simulink model "TosAeb"  (R2021a)
settings: Solver=FixedStepDiscrete  StartTime=0.0  StopTime=inf  FixedStep=0.01  RelTol=1e-3
undefined symbols (not defined in the file or in its folder): ACCModSts, AccSts1, AEB_ObjectMovingOrientation, AEB_ObjectMovingState, CN_BTCActive, CN_CurrentGearR, CN_CurrentGearR_DHT, CN_PTCActive, CN_VDCActive, CN_VSC_EspOff, CN_VSC_FCW_AEB_Error, CN_VSC_FCW_AEB_NoDispay, CN_VSC_FCW_AEB_PerfmDgdtn, CN_VSC_FCW_AEB_TempUnAvlbl, CN_VSC_VehStandstill, CNaccelAvoidMax, CNaccelAvoidMin, CNbrakePedalPress, CNcomfortStrLeftAccelMax, CNcomfortStrLeftAccelMin, CNcomfortStrRightAccelMax, CNcomfortStrRightAccelMin, CNDrvDoorOpen, CNDrvModeEco, CNDrvModeNorm, CNDrvModeOther, CNDrvModeSport, CNDrvSBRreminder, CNNull, CNobjInvalidID, CNPassDoorOpen, CNPassSBRreminder, CNPEBSAccelNegInf, CNPEBSAccelNull, CNPEBSTimeInf, CNPEBSTimeNull, CNSTOPMAX, CNSTOPNULL, CNsubjWidth, CNSwtOFF, CNSwtON, CNTTCMAX, CNTTCNULL, CNVehConfigAEBCycNone, CNVehConfigAEBNone, CNVehConfigAEBPedNone, CNVehConfigFCWNone, CNVehConfigJANone, CollisionSeverity, FALSE, FCWSnvty, JACycObjCalcInfoDefaultParams, JAPedObjCalcInfoDefaultParams, JAVehObjCalcInfoDefaultParams, latCycObjCalcInfoDefaultParams, latPedObjCalcInfoDefaultParams, longObjCalcInfoDefaultParams, ModSts, movingStatus, ObjectType ... (+1112)
libraries: simulink x3031, AEBSFunctionLib x50, Ta_Library x33, AEBSStateMachineLib x23, Mylibrary x12, simulink_extras x7, ObjSelect_lib x3, dspstat3 x2
(part 32 of 102)

== system TosAeb/FcwAebJa_Step_sys/ALG_AEBSystem/ALG_AEBController/AEB_StateMachineCalcApp/LatAEBStateMachine/pedPrefillStateMachine/ActivationCondCalc ==
  pedPrefillSuppCond : Inport
  pedPrefillSuppArb : Inport  Port=2
  pedPrefillFuncImpSuppCond : Inport  Port=3
  Compare To Constant : simulink/Logic and Bit Operations/Compare To Constant  relop===  const=FALSE  OutDataTypeStr=boolean
  Compare To Constant1 : simulink/Logic and Bit Operations/Compare To Constant  relop===  const=FALSE  OutDataTypeStr=boolean
  Compare To Constant2 : simulink/Logic and Bit Operations/Compare To Constant  relop===  const=FALSE  OutDataTypeStr=boolean
  Logical Operator : Logic  Inputs=3  AllPortsSameDT=off  OutDataTypeStr=boolean
  ActivationCondFulfilled : Outport
  wires:
  pedPrefillSuppCond/1 -> Compare To Constant/1
  pedPrefillSuppArb/1 -> Compare To Constant1/1
  pedPrefillFuncImpSuppCond/1 -> Compare To Constant2/1
  Compare To Constant1/1 -> Logical Operator/2
  Compare To Constant/1 -> Logical Operator/1
  Compare To Constant2/1 -> Logical Operator/3
  Logical Operator/1 -> ActivationCondFulfilled/1

== system TosAeb/FcwAebJa_Step_sys/ALG_AEBSystem/ALG_AEBController/AEB_StateMachineCalcApp/LatAEBStateMachine/pedPrefillStateMachine/ActiveStateAborCondCalc ==
  pedPrefillAborCond : Inport
  pedPrefillAborArb : Inport  Port=2
  pedPrefillFuncImpAborCond : Inport  Port=3
  vehBrkManeuAborCond : Inport  Port=4
  Compare To Constant{,1..3} (4 alike) : simulink/Logic and Bit Operations/Compare To Constant  relop===  const=TRUE  OutDataTypeStr=boolean
  Logical Operator : Logic  Operator=OR  Inputs=4  AllPortsSameDT=off  OutDataTypeStr=boolean
  ActiveStateAborCondFulfilled : Outport
  wires:
  Logical Operator/1 -> ActiveStateAborCondFulfilled/1
  Compare To Constant2/1 -> Logical Operator/3
  Compare To Constant/1 -> Logical Operator/1
  Compare To Constant1/1 -> Logical Operator/2
  pedPrefillFuncImpAborCond/1 -> Compare To Constant2/1
  pedPrefillAborArb/1 -> Compare To Constant1/1
  pedPrefillAborCond/1 -> Compare To Constant/1
  vehBrkManeuAborCond/1 -> Compare To Constant3/1
  Compare To Constant3/1 -> Logical Operator/4

== system TosAeb/FcwAebJa_Step_sys/ALG_AEBSystem/ALG_AEBController/AEB_StateMachineCalcApp/LatFctStsArb/BrkArb/BrkAborArb ==
  vehAEBModSts : Inport
  Bus Selector : BusSelector  OutputSignals=cycAEBfullModSts,pedAEBfullModSts
  Compare To Constant2 : simulink/Logic and Bit Operations/Compare To Constant  relop===  const=ModSts.ActiveState  OutDataTypeStr=boolean
  Compare To Constant3 : simulink/Logic and Bit Operations/Compare To Constant  relop===  const=ModSts.ActiveState  OutDataTypeStr=boolean
  Constant : Constant  Value=FALSE  OutDataTypeStr=boolean
  Logical Operator : Logic  Operator=OR  AllPortsSameDT=off  OutDataTypeStr=boolean
  cycAEBpartAborArb : Outport
  cycAEBfullAborArb : Outport  Port=2
  pedAEBfullAborArb : Outport  Port=3
  wires:
  Constant/1 -> pedAEBfullAborArb/1
  vehAEBModSts/1 -> Bus Selector/1
  Compare To Constant3/1 -> Logical Operator/2, cycAEBfullAborArb/1
  Compare To Constant2/1 -> Logical Operator/1
  Logical Operator/1 -> cycAEBpartAborArb/1
  Bus Selector/1 -> Compare To Constant2/1  "<cycAEBfullModSts>"
  Bus Selector/2 -> Compare To Constant3/1  "<pedAEBfullModSts>"

== system TosAeb/FcwAebJa_Step_sys/ALG_AEBSystem/ALG_AEBController/AEB_StateMachineCalcApp/LatFctStsArb/BrkArb/BrkActvArb ==
  cycAEBpartModStsArb : Inport
  cycAEBfullModStsArb : Inport  Port=2
  pedAEBfullModStsArb : Inport  Port=3
  Compare To Constant : simulink/Logic and Bit Operations/Compare To Constant  relop===  const=ModSts.ActiveState  OutDataTypeStr=boolean
  Compare To Constant1 : simulink/Logic and Bit Operations/Compare To Constant  relop===  const=ModSts.ActiveState  OutDataTypeStr=boolean
  Compare To Constant2 : simulink/Logic and Bit Operations/Compare To Constant  relop===  const=ModSts.ActiveState  OutDataTypeStr=boolean
  Logical Operator : Logic  Operator=OR  Inputs=3  AllPortsSameDT=off  OutDataTypeStr=boolean
  brkActvCond : Outport
  wires:
  pedAEBfullModStsArb/1 -> Compare To Constant2/1
  cycAEBfullModStsArb/1 -> Compare To Constant1/1
  cycAEBpartModStsArb/1 -> Compare To Constant/1
  Logical Operator/1 -> brkActvCond/1
  Compare To Constant/1 -> Logical Operator/1
  Compare To Constant1/1 -> Logical Operator/2
  Compare To Constant2/1 -> Logical Operator/3

== system TosAeb/FcwAebJa_Step_sys/ALG_AEBSystem/ALG_AEBController/AEB_StateMachineCalcApp/LatFctStsArb/BrkArb/BrkModStsArb ==
  vehAEBModSts : Inport
  cycAEBpartAborArb : Inport  Port=2
  cycAEBfullAborArb : Inport  Port=3
  pedAEBfullAborArb : Inport  Port=4
  Bus Selector : BusSelector  OutputSignals=cycAEBpartModSts
  Bus Selector1 : BusSelector  OutputSignals=cycAEBfullModSts
  Bus Selector2 : BusSelector  OutputSignals=pedAEBfullModSts
  Compare To Constant : simulink/Logic and Bit Operations/Compare To Constant  relop=~=  const=ModSts.ActiveState  OutDataTypeStr=boolean
  Compare To Constant1 : simulink/Logic and Bit Operations/Compare To Constant  relop===  const=FALSE  OutDataTypeStr=boolean
  Compare To Constant2 : simulink/Logic and Bit Operations/Compare To Constant  relop=~=  const=ModSts.ActiveState  OutDataTypeStr=boolean
  Compare To Constant3 : simulink/Logic and Bit Operations/Compare To Constant  relop===  const=FALSE  OutDataTypeStr=boolean
  Compare To Constant4 : simulink/Logic and Bit Operations/Compare To Constant  relop=~=  const=ModSts.ActiveState  OutDataTypeStr=boolean
  Compare To Constant5 : simulink/Logic and Bit Operations/Compare To Constant  relop===  const=FALSE  OutDataTypeStr=boolean
  Constant : Constant  Value=ModSts.StandbyState
  Constant1 : Constant  Value=ModSts.StandbyState
  Constant2 : Constant  Value=ModSts.StandbyState
  Switch{,1..5} (6 alike) : Switch  Criteria=u2 ~= 0
  cycAEBpartModStsArb : Outport
  cycAEBfullModStsArb : Outport  Port=2
  pedAEBfullModStsArb : Outport  Port=3
  wires:
  vehAEBModSts/1 -> Bus Selector/1, Bus Selector2/1, Bus Selector1/1
  Switch2/1 -> cycAEBfullModStsArb/1
  Switch3/1 -> Switch2/3
  Constant1/1 -> Switch3/3
  Compare To Constant3/1 -> Switch3/2
  cycAEBfullAborArb/1 -> Compare To Constant3/1
  Compare To Constant2/1 -> Switch2/2
  Bus Selector1/1 -> Compare To Constant2/1, Switch3/1, Switch2/1  "<cycAEBfullModSts>"
  Switch/1 -> cycAEBpartModStsArb/1
  Constant/1 -> Switch1/3
  cycAEBpartAborArb/1 -> Compare To Constant1/1
  Compare To Constant1/1 -> Switch1/2
  Switch1/1 -> Switch/3
  Bus Selector/1 -> Compare To Constant/1, Switch1/1, Switch/1  "<cycAEBpartModSts>"
  Compare To Constant/1 -> Switch/2
  Switch4/1 -> pedAEBfullModStsArb/1
  pedAEBfullAborArb/1 -> Compare To Constant5/1
  Bus Selector2/1 -> Compare To Constant4/1, Switch5/1, Switch4/1  "<pedAEBfullModSts>"
  Compare To Constant4/1 -> Switch4/2
  Compare To Constant5/1 -> Switch5/2
  Constant2/1 -> Switch5/3
  Switch5/1 -> Switch4/3

== system TosAeb/FcwAebJa_Step_sys/ALG_AEBSystem/ALG_AEBController/AEB_StateMachineCalcApp/LatFctStsArb/BrkArb/BrkSuppArb ==
  vehAEBModSts : Inport
  Bus Selector : BusSelector  OutputSignals=cycAEBfullModSts,pedAEBfullModSts
  Compare To Constant2 : simulink/Logic and Bit Operations/Compare To Constant  relop===  const=ModSts.ActiveState  OutDataTypeStr=boolean
  Compare To Constant6 : simulink/Logic and Bit Operations/Compare To Constant  relop===  const=ModSts.ActiveState  OutDataTypeStr=boolean
  Constant : Constant  Value=FALSE
  Logical Operator : Logic  Operator=OR  AllPortsSameDT=off  OutDataTypeStr=boolean
  cycAEBpartSuppArb : Outport
  cycAEBfullSuppArb : Outport  Port=2
  pedAEBfullSuppArb : Outport  Port=3
  wires:
  Constant/1 -> pedAEBfullSuppArb/1
  Compare To Constant2/1 -> Logical Operator/2, cycAEBfullSuppArb/1
  Compare To Constant6/1 -> Logical Operator/1
  vehAEBModSts/1 -> Bus Selector/1
  Logical Operator/1 -> cycAEBpartSuppArb/1
  Bus Selector/1 -> Compare To Constant6/1  "<cycAEBfullModSts>"
  Bus Selector/2 -> Compare To Constant2/1  "<pedAEBfullModSts>"

== system TosAeb/FcwAebJa_Step_sys/ALG_AEBSystem/ALG_AEBController/AEB_StateMachineCalcApp/LatFctStsArb/BrkReadyArb/BrkReadyAborArb ==
  brkActvCond : Inport
  latAEBModSts : Inport  Port=2
  Bus Selector : BusSelector  OutputSignals=pedPrefillModSts
  Compare To Constant : simulink/Logic and Bit Operations/Compare To Constant  relop===  const=TRUE  OutDataTypeStr=boolean
  Compare To Constant2 : simulink/Logic and Bit Operations/Compare To Constant  relop===  const=ModSts.ActiveState  OutDataTypeStr=boolean
  Constant : Constant  Value=FALSE  OutDataTypeStr=boolean
  Logical Operator : Logic  Operator=OR  AllPortsSameDT=off  OutDataTypeStr=boolean
  pedPrefillAborArb : Outport
  cycPrefillAborArb : Outport  Port=2
  pedABAAborArb : Outport  Port=3
  wires:
  brkActvCond/1 -> Compare To Constant/1
  Constant/1 -> pedABAAborArb/1
  latAEBModSts/1 -> Bus Selector/1
  Bus Selector/1 -> Compare To Constant2/1  "<pedPrefillModSts>"
  Compare To Constant/1 -> Logical Operator/1, pedPrefillAborArb/1
  Compare To Constant2/1 -> Logical Operator/2
  Logical Operator/1 -> cycPrefillAborArb/1

== system TosAeb/FcwAebJa_Step_sys/ALG_AEBSystem/ALG_AEBController/AEB_StateMachineCalcApp/LatFctStsArb/BrkReadyArb/BrkReadyActvArb ==
  prefillModStsArb : Inport
  ABAModStsArb : Inport  Port=2
  pedABAModStsArb : Inport  Port=3
  Compare To Constant : simulink/Logic and Bit Operations/Compare To Constant  relop===  const=ModSts.ActiveState  OutDataTypeStr=boolean
  Compare To Constant1 : simulink/Logic and Bit Operations/Compare To Constant  relop===  const=ModSts.ActiveState  OutDataTypeStr=boolean
  Compare To Constant2 : simulink/Logic and Bit Operations/Compare To Constant  relop===  const=ModSts.ActiveState  OutDataTypeStr=boolean
  Logical Operator : Logic  Operator=OR  Inputs=3  AllPortsSameDT=off  OutDataTypeStr=boolean
  brkReadyActv : Outport
  wires:
  ABAModStsArb/1 -> Compare To Constant1/1
  Logical Operator/1 -> brkReadyActv/1
  prefillModStsArb/1 -> Compare To Constant/1
  pedABAModStsArb/1 -> Compare To Constant2/1
  Compare To Constant/1 -> Logical Operator/1
  Compare To Constant1/1 -> Logical Operator/2
  Compare To Constant2/1 -> Logical Operator/3

== system TosAeb/FcwAebJa_Step_sys/ALG_AEBSystem/ALG_AEBController/AEB_StateMachineCalcApp/LatFctStsArb/BrkReadyArb/BrkReadyModStsArb ==
  vehAEBModSts : Inport
  pedPrefillAborArb : Inport  Port=2
  cycPrefillAborArb : Inport  Port=3
  pedABAAborArb : Inport  Port=4
  Bus Selector : BusSelector  OutputSignals=pedPrefillModSts
  Bus Selector1 : BusSelector  OutputSignals=cycPrefillModSts
  Bus Selector2 : BusSelector  OutputSignals=pedABAModSts
  Compare To Constant1 : simulink/Logic and Bit Operations/Compare To Constant  relop=~=  const=ModSts.ActiveState  OutDataTypeStr=boolean
  Compare To Constant2 : simulink/Logic and Bit Operations/Compare To Constant  relop=~=  const=ModSts.ActiveState  OutDataTypeStr=boolean
  Compare To Constant3 : simulink/Logic and Bit Operations/Compare To Constant  relop===  const=FALSE  OutDataTypeStr=boolean
  Compare To Constant4 : simulink/Logic and Bit Operations/Compare To Constant  relop=~=  const=ModSts.ActiveState  OutDataTypeStr=boolean
  Compare To Constant5 : simulink/Logic and Bit Operations/Compare To Constant  relop===  const=FALSE  OutDataTypeStr=boolean
  Compare To Constant6 : simulink/Logic and Bit Operations/Compare To Constant  relop===  const=FALSE  OutDataTypeStr=boolean
  Constant1 : Constant  Value=ModSts.StandbyState
  Constant2 : Constant  Value=ModSts.StandbyState
  Constant3 : Constant  Value=ModSts.StandbyState
  Switch{1..6} (6 alike) : Switch  Criteria=u2 ~= 0
  pedPrefillModStsArb : Outport
  cycPrefillModStsArb : Outport  Port=2
  pedABAModStsArb : Outport  Port=3
  wires:
  Switch2/1 -> cycPrefillModStsArb/1  "cycPrefillModStsArb"
  Switch3/1 -> Switch2/3
  Constant1/1 -> Switch3/3
  Compare To Constant3/1 -> Switch3/2
  cycPrefillAborArb/1 -> Compare To Constant3/1
  Compare To Constant2/1 -> Switch2/2
  Bus Selector1/1 -> Compare To Constant2/1, Switch2/1, Switch3/1  "<cycPrefillModSts>"
  Switch1/1 -> pedPrefillModStsArb/1  "pedPrefillModStsArb"
  vehAEBModSts/1 -> Bus Selector/1, Bus Selector2/1, Bus Selector1/1
  Bus Selector2/1 -> Switch5/1, Switch4/1, Compare To Constant4/1  "<pedABAModSts>"
  Compare To Constant4/1 -> Switch4/2
  pedABAAborArb/1 -> Compare To Constant5/1
  Compare To Constant5/1 -> Switch5/2
  Constant2/1 -> Switch5/3
  Switch5/1 -> Switch4/3
  Switch4/1 -> pedABAModStsArb/1
  pedPrefillAborArb/1 -> Compare To Constant6/1
  Compare To Constant1/1 -> Switch1/2
  Constant3/1 -> Switch6/3
  Switch6/1 -> Switch1/3
  Bus Selector/1 -> Switch1/1, Switch6/1, Compare To Constant1/1  "<pedPrefillModSts>"
  Compare To Constant6/1 -> Switch6/2

== system TosAeb/FcwAebJa_Step_sys/ALG_AEBSystem/ALG_AEBController/AEB_StateMachineCalcApp/LatFctStsArb/BrkReadyArb/BrkReadySuppArb ==
  latAEBModSts : Inport
  brkActvCond : Inport  Port=2
  Bus Selector : BusSelector  OutputSignals=pedPrefillModSts
  Compare To Constant : simulink/Logic and Bit Operations/Compare To Constant  relop===  const=TRUE  OutDataTypeStr=boolean
  Compare To Constant1 : simulink/Logic and Bit Operations/Compare To Constant  relop===  const=ModSts.ActiveState  OutDataTypeStr=boolean
  Constant : Constant  Value=FALSE  OutDataTypeStr=boolean
  Logical Operator : Logic  Operator=OR  AllPortsSameDT=off  OutDataTypeStr=boolean
  cycPrefillSuppArb : Outport
  pedPrefillSuppArb : Outport  Port=2
  pedABASuppArb : Outport  Port=3
  wires:
  Constant/1 -> pedABASuppArb/1
  brkActvCond/1 -> Compare To Constant/1
  latAEBModSts/1 -> Bus Selector/1
  Bus Selector/1 -> Compare To Constant1/1  "<pedPrefillModSts>"
  Compare To Constant1/1 -> Logical Operator/1
  Compare To Constant/1 -> Logical Operator/2, pedPrefillSuppArb/1
  Logical Operator/1 -> cycPrefillSuppArb/1

== system TosAeb/FcwAebJa_Step_sys/ALG_AEBSystem/ALG_AEBController/AEB_StateMachineCalcApp/LatFctStsArb/WarningArb/WarningAborArb ==
  brkActvCond : Inport
  Compare To Constant5 : simulink/Logic and Bit Operations/Compare To Constant  relop===  const=TRUE  OutDataTypeStr=boolean
  pedPreWarningAborArb : Outport
  wires:
  Compare To Constant5/1 -> pedPreWarningAborArb/1
  brkActvCond/1 -> Compare To Constant5/1

== system TosAeb/FcwAebJa_Step_sys/ALG_AEBSystem/ALG_AEBController/AEB_StateMachineCalcApp/LatFctStsArb/WarningArb/WarningActvArb ==
  pedPreWarningModStsArb : Inport
  Compare To Constant : simulink/Logic and Bit Operations/Compare To Constant  relop===  const=ModSts.ActiveState  OutDataTypeStr=boolean
  pedPreWarningActvCond : Outport
  wires:
  pedPreWarningModStsArb/1 -> Compare To Constant/1
  Compare To Constant/1 -> pedPreWarningActvCond/1

== system TosAeb/FcwAebJa_Step_sys/ALG_AEBSystem/ALG_AEBController/AEB_StateMachineCalcApp/LatFctStsArb/WarningArb/WarningModStsArb ==
  vehAEBModSts : Inport
  pedPreWarningAborArb : Inport  Port=2
  Bus Selector : BusSelector  OutputSignals=pedPreWarningModSts
  Compare To Constant : simulink/Logic and Bit Operations/Compare To Constant  relop=~=  const=ModSts.ActiveState  OutDataTypeStr=boolean
  Compare To Constant1 : simulink/Logic and Bit Operations/Compare To Constant  relop===  const=FALSE  OutDataTypeStr=boolean
  Constant : Constant  Value=ModSts.StandbyState
  Switch : Switch  Criteria=u2 ~= 0
  Switch1 : Switch  Criteria=u2 ~= 0
  pedPreWarningModStsArb : Outport
  wires:
  vehAEBModSts/1 -> Bus Selector/1
  Bus Selector/1 -> Switch/1, Switch1/1, Compare To Constant/1  "<pedPreWarningModSts>"
  Compare To Constant/1 -> Switch/2
  Switch1/1 -> Switch/3
  Compare To Constant1/1 -> Switch1/2
  pedPreWarningAborArb/1 -> Compare To Constant1/1
  Constant/1 -> Switch1/3
  Switch/1 -> pedPreWarningModStsArb/1

== system TosAeb/FcwAebJa_Step_sys/ALG_AEBSystem/ALG_AEBController/AEB_StateMachineCalcApp/LatFctStsArb/WarningArb/WarningSuppArb ==
  brkActvCond : Inport
  Compare To Constant4 : simulink/Logic and Bit Operations/Compare To Constant  relop===  const=TRUE  OutDataTypeStr=boolean
  pedPrewarningSuppArb : Outport
  wires:
  Compare To Constant4/1 -> pedPrewarningSuppArb/1
  brkActvCond/1 -> Compare To Constant4/1

== system TosAeb/FcwAebJa_Step_sys/ALG_AEBSystem/ALG_AEBController/AEB_StateMachineCalcApp/VehAEBStateMachine/ABAStateMachine/AEBFuncFaultMap ==
  FCW_AEB_VehFctSts : Inport
  Compare To Constant : simulink/Logic and Bit Operations/Compare To Constant  relop===  const=CN_VSC_FCW_AEB_Error  OutDataTypeStr=boolean
  Fault : Outport
  wires:
  FCW_AEB_VehFctSts/1 -> Compare To Constant/1
  Compare To Constant/1 -> Fault/1

== system TosAeb/FcwAebJa_Step_sys/ALG_AEBSystem/ALG_AEBController/AEB_StateMachineCalcApp/VehAEBStateMachine/ABAStateMachine/ActivationCondCalc ==
  vehABASuppCond : Inport
  ABABrkReadySuppArb : Inport  Port=2
  vehABAFuncImpSuppCond : Inport  Port=3
  Compare To Constant : simulink/Logic and Bit Operations/Compare To Constant  relop===  const=FALSE  OutDataTypeStr=boolean
  Compare To Constant1 : simulink/Logic and Bit Operations/Compare To Constant  relop===  const=FALSE  OutDataTypeStr=boolean
  Compare To Constant2 : simulink/Logic and Bit Operations/Compare To Constant  relop===  const=FALSE  OutDataTypeStr=boolean
  Logical Operator : Logic  Inputs=3  AllPortsSameDT=off  OutDataTypeStr=boolean
  ActivationCondFulfilled : Outport
  wires:
  vehABASuppCond/1 -> Compare To Constant/1
  ABABrkReadySuppArb/1 -> Compare To Constant1/1
  vehABAFuncImpSuppCond/1 -> Compare To Constant2/1
  Compare To Constant1/1 -> Logical Operator/2
  Compare To Constant/1 -> Logical Operator/1
  Compare To Constant2/1 -> Logical Operator/3
  Logical Operator/1 -> ActivationCondFulfilled/1

== system TosAeb/FcwAebJa_Step_sys/ALG_AEBSystem/ALG_AEBController/AEB_StateMachineCalcApp/VehAEBStateMachine/ABAStateMachine/ActiveStateAborCondCalc ==
  vehABAAborCond : Inport
  ABABrkReadyAborArb : Inport  Port=2
  vehABAFuncImpAborCond : Inport  Port=3
  ABAFuncManeuAborCond : Inport  Port=4
  Compare To Constant{,1..3} (4 alike) : simulink/Logic and Bit Operations/Compare To Constant  relop===  const=TRUE  OutDataTypeStr=boolean
  Logical Operator : Logic  Operator=OR  Inputs=4  AllPortsSameDT=off  OutDataTypeStr=boolean
  ActiveStateAborCondFulfilled : Outport
  wires:
  Logical Operator/1 -> ActiveStateAborCondFulfilled/1
  Compare To Constant2/1 -> Logical Operator/3
  Compare To Constant/1 -> Logical Operator/1
  Compare To Constant1/1 -> Logical Operator/2
  vehABAFuncImpAborCond/1 -> Compare To Constant2/1
  ABABrkReadyAborArb/1 -> Compare To Constant1/1
  vehABAAborCond/1 -> Compare To Constant/1
  ABAFuncManeuAborCond/1 -> Compare To Constant3/1
  Compare To Constant3/1 -> Logical Operator/4

== system TosAeb/FcwAebJa_Step_sys/ALG_AEBSystem/ALG_AEBController/AEB_StateMachineCalcApp/VehAEBStateMachine/AEBfullStateMachine/AEBFuncFaultMap ==
  FCW_AEB_VehFctSts : Inport
  Compare To Constant : simulink/Logic and Bit Operations/Compare To Constant  relop===  const=CN_VSC_FCW_AEB_Error  OutDataTypeStr=boolean
  Fault : Outport
  wires:
  FCW_AEB_VehFctSts/1 -> Compare To Constant/1
  Compare To Constant/1 -> Fault/1

== system TosAeb/FcwAebJa_Step_sys/ALG_AEBSystem/ALG_AEBController/AEB_StateMachineCalcApp/VehAEBStateMachine/AEBfullStateMachine/ActivationCondCalc ==
  vehAEBfullSuppCond : Inport
  AEBfullSuppArb : Inport  Port=2
  vehAEBfullFuncImpSuppCond : Inport  Port=3
  Compare To Constant : simulink/Logic and Bit Operations/Compare To Constant  relop===  const=FALSE  OutDataTypeStr=boolean
  Compare To Constant1 : simulink/Logic and Bit Operations/Compare To Constant  relop===  const=FALSE  OutDataTypeStr=boolean
  Compare To Constant2 : simulink/Logic and Bit Operations/Compare To Constant  relop===  const=FALSE  OutDataTypeStr=boolean
  Logical Operator : Logic  Inputs=3  AllPortsSameDT=off  OutDataTypeStr=boolean
  ActivationCondFulfilled : Outport
  wires:
  vehAEBfullSuppCond/1 -> Compare To Constant/1
  AEBfullSuppArb/1 -> Compare To Constant1/1
  vehAEBfullFuncImpSuppCond/1 -> Compare To Constant2/1
  Compare To Constant1/1 -> Logical Operator/2
  Compare To Constant/1 -> Logical Operator/1
  Compare To Constant2/1 -> Logical Operator/3
  Logical Operator/1 -> ActivationCondFulfilled/1

== system TosAeb/FcwAebJa_Step_sys/ALG_AEBSystem/ALG_AEBController/AEB_StateMachineCalcApp/VehAEBStateMachine/AEBfullStateMachine/ActiveStateAborCondCalc ==
  AEBfullAborCondNoStandstill : Inport
  AEBfullAborArb : Inport  Port=2
  vehAEBfullFuncImpAborCond : Inport  Port=3
  vehBrkManeuAborCond : Inport  Port=4
  Compare To Constant{,1..3} (4 alike) : simulink/Logic and Bit Operations/Compare To Constant  relop===  const=TRUE  OutDataTypeStr=boolean
  Logical Operator : Logic  Operator=OR  Inputs=4  AllPortsSameDT=off  OutDataTypeStr=boolean
  ActiveStateAborCondFulfilled : Outport
  wires:
  Logical Operator/1 -> ActiveStateAborCondFulfilled/1
  Compare To Constant2/1 -> Logical Operator/3
  Compare To Constant/1 -> Logical Operator/1
  Compare To Constant1/1 -> Logical Operator/2
  vehAEBfullFuncImpAborCond/1 -> Compare To Constant2/1
  AEBfullAborArb/1 -> Compare To Constant1/1
  AEBfullAborCondNoStandstill/1 -> Compare To Constant/1
  vehBrkManeuAborCond/1 -> Compare To Constant3/1
  Compare To Constant3/1 -> Logical Operator/4

== system TosAeb/FcwAebJa_Step_sys/ALG_AEBSystem/ALG_AEBController/AEB_StateMachineCalcApp/VehAEBStateMachine/AEBfullStateMachine/StandstillAborCondCalc ==
  AEBfullAborCondStandstill : Inport
  AEBfullAborArb : Inport  Port=2
  vehAEBfullFuncImpAborCond : Inport  Port=3
  vehBrkManeuAborCond : Inport  Port=4
  Compare To Constant{,1..3} (4 alike) : simulink/Logic and Bit Operations/Compare To Constant  relop===  const=TRUE  OutDataTypeStr=boolean
  Logical Operator : Logic  Operator=OR  Inputs=4  AllPortsSameDT=off  OutDataTypeStr=boolean
  StandstillAborCondFulfilled : Outport
  wires:
  Compare To Constant3/1 -> Logical Operator/4
  vehBrkManeuAborCond/1 -> Compare To Constant3/1
  AEBfullAborCondStandstill/1 -> Compare To Constant/1
  AEBfullAborArb/1 -> Compare To Constant1/1
  vehAEBfullFuncImpAborCond/1 -> Compare To Constant2/1
  Compare To Constant1/1 -> Logical Operator/2
  Compare To Constant/1 -> Logical Operator/1
  Compare To Constant2/1 -> Logical Operator/3
  Logical Operator/1 -> StandstillAborCondFulfilled/1

== system TosAeb/FcwAebJa_Step_sys/ALG_AEBSystem/ALG_AEBController/AEB_StateMachineCalcApp/VehAEBStateMachine/AEBpartStateMachine/AEBFuncFaultMap ==
  FCW_AEB_VehFctSts : Inport
  Compare To Constant : simulink/Logic and Bit Operations/Compare To Constant  relop===  const=CN_VSC_FCW_AEB_Error  OutDataTypeStr=boolean
  Fault : Outport
  wires:
  FCW_AEB_VehFctSts/1 -> Compare To Constant/1
  Compare To Constant/1 -> Fault/1

== system TosAeb/FcwAebJa_Step_sys/ALG_AEBSystem/ALG_AEBController/AEB_StateMachineCalcApp/VehAEBStateMachine/AEBpartStateMachine/ActivationCondCalc ==
  vehAEBpartSuppCond : Inport
  AEBpartSuppArb : Inport  Port=2
  vehAEBpartFuncImpSuppCond : Inport  Port=3
  Compare To Constant : simulink/Logic and Bit Operations/Compare To Constant  relop===  const=FALSE  OutDataTypeStr=boolean
  Compare To Constant1 : simulink/Logic and Bit Operations/Compare To Constant  relop===  const=FALSE  OutDataTypeStr=boolean
  Compare To Constant2 : simulink/Logic and Bit Operations/Compare To Constant  relop===  const=FALSE  OutDataTypeStr=boolean
  Logical Operator : Logic  Inputs=3  AllPortsSameDT=off  OutDataTypeStr=boolean
  ActivationCondFulfilled : Outport
  wires:
  Logical Operator/1 -> ActivationCondFulfilled/1
  Compare To Constant2/1 -> Logical Operator/3
  Compare To Constant/1 -> Logical Operator/1
  Compare To Constant1/1 -> Logical Operator/2
  vehAEBpartFuncImpSuppCond/1 -> Compare To Constant2/1
  AEBpartSuppArb/1 -> Compare To Constant1/1
  vehAEBpartSuppCond/1 -> Compare To Constant/1

== system TosAeb/FcwAebJa_Step_sys/ALG_AEBSystem/ALG_AEBController/AEB_StateMachineCalcApp/VehAEBStateMachine/AEBpartStateMachine/ActiveStateAborCondCalc ==
  vehAEBpartAborCondNoStandstill : Inport
  AEBpartSuppArb : Inport  Port=2
  vehAEBpartFuncImpAborCond : Inport  Port=3
  vehBrkManeuAborCond : Inport  Port=4
  Compare To Constant{,1..3} (4 alike) : simulink/Logic and Bit Operations/Compare To Constant  relop===  const=TRUE  OutDataTypeStr=boolean
  Logical Operator : Logic  Operator=OR  Inputs=4  AllPortsSameDT=off  OutDataTypeStr=boolean
  ActiveStateAborCondFulfilled : Outport
  wires:
  Compare To Constant3/1 -> Logical Operator/4
  vehBrkManeuAborCond/1 -> Compare To Constant3/1  "vehBrkManeuAborCond"
  vehAEBpartAborCondNoStandstill/1 -> Compare To Constant/1  "vehAEBpartAborCondNoStandstill"
  AEBpartSuppArb/1 -> Compare To Constant1/1  "AEBpartSuppArb"
  vehAEBpartFuncImpAborCond/1 -> Compare To Constant2/1  "vehAEBpartFuncImpAborCond"
  Compare To Constant1/1 -> Logical Operator/2
  Compare To Constant/1 -> Logical Operator/1
  Compare To Constant2/1 -> Logical Operator/3
  Logical Operator/1 -> ActiveStateAborCondFulfilled/1  "ActiveStateAborCondFulfilled"

== system TosAeb/FcwAebJa_Step_sys/ALG_AEBSystem/ALG_AEBController/AEB_StateMachineCalcApp/VehAEBStateMachine/AEBpartStateMachine/StandstillAborCondCalc ==
  ABEfullAborCondStandstill : Inport
  BrkFuncAborCond : Inport  Port=2
  brkImpAborCond : Inport  Port=3
  brkImpOpcAborCond : Inport  Port=4
  Compare To Constant{,1..3} (4 alike) : simulink/Logic and Bit Operations/Compare To Constant  relop===  const=TRUE  OutDataTypeStr=boolean
  Logical Operator : Logic  Operator=OR  Inputs=4  AllPortsSameDT=off  OutDataTypeStr=boolean
  StandstillAborCondFulfilled : Outport
  wires:
  Logical Operator/1 -> StandstillAborCondFulfilled/1  "StandstillAborCondFulfilled"
  Compare To Constant2/1 -> Logical Operator/3
  Compare To Constant/1 -> Logical Operator/1
  Compare To Constant1/1 -> Logical Operator/2
  brkImpAborCond/1 -> Compare To Constant2/1  "brkImpAborCond"
  BrkFuncAborCond/1 -> Compare To Constant1/1  "BrkFuncAborCond"
  ABEfullAborCondStandstill/1 -> Compare To Constant/1  "ABEfullAborCondStandstill"
  brkImpOpcAborCond/1 -> Compare To Constant3/1  "brkImpOpcAborCond"
  Compare To Constant3/1 -> Logical Operator/4

== system TosAeb/FcwAebJa_Step_sys/ALG_AEBSystem/ALG_AEBController/AEB_StateMachineCalcApp/VehAEBStateMachine/ActuteWarningStateMachine/AEBFuncFaultMap ==
  FCW_AEB_VehFctSts : Inport
  Compare To Constant : simulink/Logic and Bit Operations/Compare To Constant  relop===  const=CN_VSC_FCW_AEB_Error  OutDataTypeStr=boolean
  Fault : Outport
  wires:
  FCW_AEB_VehFctSts/1 -> Compare To Constant/1
  Compare To Constant/1 -> Fault/1

== system TosAeb/FcwAebJa_Step_sys/ALG_AEBSystem/ALG_AEBController/AEB_StateMachineCalcApp/VehAEBStateMachine/ActuteWarningStateMachine/ActivationCondCalc ==
  vehAcuteWarningSuppCond : Inport
  acuteWarningSuppArb : Inport  Port=2
  vehAcuteWarningFuncImpSuppCond : Inport  Port=3
  Compare To Constant : simulink/Logic and Bit Operations/Compare To Constant  relop===  const=FALSE  OutDataTypeStr=boolean
  Compare To Constant1 : simulink/Logic and Bit Operations/Compare To Constant  relop===  const=FALSE  OutDataTypeStr=boolean
  Compare To Constant2 : simulink/Logic and Bit Operations/Compare To Constant  relop===  const=FALSE  OutDataTypeStr=boolean
  Logical Operator : Logic  Inputs=3  AllPortsSameDT=off  OutDataTypeStr=boolean
  acuteWarningActivationCondFulfilled : Outport
  wires:
  vehAcuteWarningSuppCond/1 -> Compare To Constant/1
  acuteWarningSuppArb/1 -> Compare To Constant1/1
  vehAcuteWarningFuncImpSuppCond/1 -> Compare To Constant2/1
  Compare To Constant1/1 -> Logical Operator/2
  Compare To Constant/1 -> Logical Operator/1
  Compare To Constant2/1 -> Logical Operator/3
  Logical Operator/1 -> acuteWarningActivationCondFulfilled/1

== system TosAeb/FcwAebJa_Step_sys/ALG_AEBSystem/ALG_AEBController/AEB_StateMachineCalcApp/VehAEBStateMachine/ActuteWarningStateMachine/ActiveStateAborCondCalc ==
  vehAcuteWarningAborCond : Inport
  acuteWarningAborArb : Inport  Port=2
  vehAcuteWarningFuncImpAborCond : Inport  Port=3
  acuteWarningFuncManeuAborCond : Inport  Port=4
  Compare To Constant{,1..3} (4 alike) : simulink/Logic and Bit Operations/Compare To Constant  relop===  const=TRUE  OutDataTypeStr=boolean
  Logical Operator : Logic  Operator=OR  Inputs=4  AllPortsSameDT=off  OutDataTypeStr=boolean
  ActiveStateAborCondFulfilled : Outport
  wires:
  Logical Operator/1 -> ActiveStateAborCondFulfilled/1
  Compare To Constant2/1 -> Logical Operator/3
  Compare To Constant/1 -> Logical Operator/1
  Compare To Constant1/1 -> Logical Operator/2
  vehAcuteWarningFuncImpAborCond/1 -> Compare To Constant2/1
  acuteWarningAborArb/1 -> Compare To Constant1/1
  vehAcuteWarningAborCond/1 -> Compare To Constant/1
  acuteWarningFuncManeuAborCond/1 -> Compare To Constant3/1
  Compare To Constant3/1 -> Logical Operator/4

== system TosAeb/FcwAebJa_Step_sys/ALG_AEBSystem/ALG_AEBController/AEB_StateMachineCalcApp/VehAEBStateMachine/EBAStateMachine/AEBFuncFaultMap ==
  FCW_AEB_VehFctSts : Inport
  Compare To Constant : simulink/Logic and Bit Operations/Compare To Constant  relop===  const=CN_VSC_FCW_AEB_Error  OutDataTypeStr=boolean
  Fault : Outport
  wires:
  FCW_AEB_VehFctSts/1 -> Compare To Constant/1
  Compare To Constant/1 -> Fault/1

== system TosAeb/FcwAebJa_Step_sys/ALG_AEBSystem/ALG_AEBController/AEB_StateMachineCalcApp/VehAEBStateMachine/EBAStateMachine/ActivationCondCalc ==
  vehEBASuppCond : Inport
  EBASuppArb : Inport  Port=2
  vehEBAFuncImpSuppCond : Inport  Port=3
  Compare To Constant : simulink/Logic and Bit Operations/Compare To Constant  relop===  const=FALSE  OutDataTypeStr=boolean
  Compare To Constant1 : simulink/Logic and Bit Operations/Compare To Constant  relop===  const=FALSE  OutDataTypeStr=boolean
  Compare To Constant2 : simulink/Logic and Bit Operations/Compare To Constant  relop===  const=FALSE  OutDataTypeStr=boolean
  Logical Operator : Logic  Inputs=3  AllPortsSameDT=off  OutDataTypeStr=boolean
  ActivationCondFulfilled : Outport
  wires:
  vehEBASuppCond/1 -> Compare To Constant/1
  EBASuppArb/1 -> Compare To Constant1/1
  vehEBAFuncImpSuppCond/1 -> Compare To Constant2/1
  Compare To Constant1/1 -> Logical Operator/2
  Compare To Constant/1 -> Logical Operator/1
  Compare To Constant2/1 -> Logical Operator/3
  Logical Operator/1 -> ActivationCondFulfilled/1

== system TosAeb/FcwAebJa_Step_sys/ALG_AEBSystem/ALG_AEBController/AEB_StateMachineCalcApp/VehAEBStateMachine/EBAStateMachine/ActiveStateAborCondCalc ==
  EBAAborCondNoStandstill : Inport
  EBAAborArb : Inport  Port=2
  vehEBAFuncImpAborCond : Inport  Port=3
  vehBrkManeuAborCond : Inport  Port=4
  Compare To Constant{,1..3} (4 alike) : simulink/Logic and Bit Operations/Compare To Constant  relop===  const=TRUE  OutDataTypeStr=boolean
  Logical Operator : Logic  Operator=OR  Inputs=4  AllPortsSameDT=off  OutDataTypeStr=boolean
  ActiveStateAborCondFulfilled : Outport
  wires:
  Logical Operator/1 -> ActiveStateAborCondFulfilled/1
  Compare To Constant2/1 -> Logical Operator/3
  Compare To Constant/1 -> Logical Operator/1
  Compare To Constant1/1 -> Logical Operator/2
  vehEBAFuncImpAborCond/1 -> Compare To Constant2/1
  EBAAborArb/1 -> Compare To Constant1/1
  EBAAborCondNoStandstill/1 -> Compare To Constant/1
  vehBrkManeuAborCond/1 -> Compare To Constant3/1
  Compare To Constant3/1 -> Logical Operator/4

== system TosAeb/FcwAebJa_Step_sys/ALG_AEBSystem/ALG_AEBController/AEB_StateMachineCalcApp/VehAEBStateMachine/EBAStateMachine/StandstillAborCondCalc ==
  EBAAborCondStandstill : Inport
  EBAAborArb : Inport  Port=2
  vehEBAFuncImpAborCond : Inport  Port=3
  vehBrkManeuAborCond : Inport  Port=4
  Compare To Constant{,1..3} (4 alike) : simulink/Logic and Bit Operations/Compare To Constant  relop===  const=TRUE  OutDataTypeStr=boolean
  Logical Operator : Logic  Operator=OR  Inputs=4  AllPortsSameDT=off  OutDataTypeStr=boolean
  StandstillAborCondFulfilled : Outport
  wires:
  Compare To Constant3/1 -> Logical Operator/4
  vehBrkManeuAborCond/1 -> Compare To Constant3/1
  EBAAborCondStandstill/1 -> Compare To Constant/1
  EBAAborArb/1 -> Compare To Constant1/1
  vehEBAFuncImpAborCond/1 -> Compare To Constant2/1
  Compare To Constant1/1 -> Logical Operator/2
  Compare To Constant/1 -> Logical Operator/1
  Compare To Constant2/1 -> Logical Operator/3
  Logical Operator/1 -> StandstillAborCondFulfilled/1

== system TosAeb/FcwAebJa_Step_sys/ALG_AEBSystem/ALG_AEBController/AEB_StateMachineCalcApp/VehAEBStateMachine/LatentWarningStateMachine/AEBFuncFaultMap ==
  FCW_AEB_VehFctSts : Inport
  Compare To Constant : simulink/Logic and Bit Operations/Compare To Constant  relop===  const=CN_VSC_FCW_AEB_Error  OutDataTypeStr=boolean
  Fault : Outport
  wires:
  FCW_AEB_VehFctSts/1 -> Compare To Constant/1
  Compare To Constant/1 -> Fault/1

== system TosAeb/FcwAebJa_Step_sys/ALG_AEBSystem/ALG_AEBController/AEB_StateMachineCalcApp/VehAEBStateMachine/LatentWarningStateMachine/ActivationCondCalc ==
  vehLatentWarningSuppCond : Inport
  latentWarningSuppArb : Inport  Port=2
  vehLatentWarningFuncImpSuppCond : Inport  Port=3
  Compare To Constant : simulink/Logic and Bit Operations/Compare To Constant  relop===  const=FALSE  OutDataTypeStr=boolean
  Compare To Constant1 : simulink/Logic and Bit Operations/Compare To Constant  relop===  const=FALSE  OutDataTypeStr=boolean
  Compare To Constant2 : simulink/Logic and Bit Operations/Compare To Constant  relop===  const=FALSE  OutDataTypeStr=boolean
  Logical Operator : Logic  Inputs=3  AllPortsSameDT=off  OutDataTypeStr=boolean
  ActivationCondFulfilled : Outport
  wires:
  vehLatentWarningSuppCond/1 -> Compare To Constant/1
  latentWarningSuppArb/1 -> Compare To Constant1/1
  vehLatentWarningFuncImpSuppCond/1 -> Compare To Constant2/1
  Compare To Constant1/1 -> Logical Operator/2
  Compare To Constant/1 -> Logical Operator/1
  Compare To Constant2/1 -> Logical Operator/3
  Logical Operator/1 -> ActivationCondFulfilled/1

== system TosAeb/FcwAebJa_Step_sys/ALG_AEBSystem/ALG_AEBController/AEB_StateMachineCalcApp/VehAEBStateMachine/LatentWarningStateMachine/ActiveStateAborCondCalc ==
  vehLatentWarningAborCond : Inport
  latentWarningAbor : Inport  Port=2
  Compare To Constant : simulink/Logic and Bit Operations/Compare To Constant  relop===  const=TRUE  OutDataTypeStr=boolean
  Compare To Constant1 : simulink/Logic and Bit Operations/Compare To Constant  relop===  const=TRUE  OutDataTypeStr=boolean
  Logical Operator : Logic  Operator=OR  AllPortsSameDT=off  OutDataTypeStr=boolean
  ActiveStateAborCondFulfilled : Outport
  wires:
  vehLatentWarningAborCond/1 -> Compare To Constant/1
  latentWarningAbor/1 -> Compare To Constant1/1
  Compare To Constant1/1 -> Logical Operator/2
  Compare To Constant/1 -> Logical Operator/1
  Logical Operator/1 -> ActiveStateAborCondFulfilled/1

== system TosAeb/FcwAebJa_Step_sys/ALG_AEBSystem/ALG_AEBController/AEB_StateMachineCalcApp/VehAEBStateMachine/PreWarningStateMachine/AEBFuncFaultMap ==
  FCW_AEB_VehFctSts : Inport
  Compare To Constant : simulink/Logic and Bit Operations/Compare To Constant  relop===  const=CN_VSC_FCW_AEB_Error  OutDataTypeStr=boolean
  Fault : Outport
  wires:
  FCW_AEB_VehFctSts/1 -> Compare To Constant/1
  Compare To Constant/1 -> Fault/1

== system TosAeb/FcwAebJa_Step_sys/ALG_AEBSystem/ALG_AEBController/AEB_StateMachineCalcApp/VehAEBStateMachine/PreWarningStateMachine/ActivationCondCalc ==
  vehPreWarningSuppCond : Inport
  preWarningSuppArb : Inport  Port=2
  vehPreWarningFuncImpSuppCond : Inport  Port=3
  Compare To Constant : simulink/Logic and Bit Operations/Compare To Constant  relop===  const=FALSE  OutDataTypeStr=boolean
  Compare To Constant1 : simulink/Logic and Bit Operations/Compare To Constant  relop===  const=FALSE  OutDataTypeStr=boolean
  Compare To Constant2 : simulink/Logic and Bit Operations/Compare To Constant  relop===  const=FALSE  OutDataTypeStr=boolean
  Logical Operator : Logic  Inputs=3  AllPortsSameDT=off  OutDataTypeStr=boolean
  vehPreWarningActivationCondFulfilled : Outport
  wires:
  Logical Operator/1 -> vehPreWarningActivationCondFulfilled/1
  Compare To Constant2/1 -> Logical Operator/3
  Compare To Constant/1 -> Logical Operator/1
  Compare To Constant1/1 -> Logical Operator/2
  vehPreWarningFuncImpSuppCond/1 -> Compare To Constant2/1
  preWarningSuppArb/1 -> Compare To Constant1/1
  vehPreWarningSuppCond/1 -> Compare To Constant/1

== system TosAeb/FcwAebJa_Step_sys/ALG_AEBSystem/ALG_AEBController/AEB_StateMachineCalcApp/VehAEBStateMachine/PreWarningStateMachine/ActiveStateAborCondCalc ==
  vehPreWarningAborCond : Inport
  preWarningSuppArb : Inport  Port=2
  vehPreWarningFuncImpAborCond : Inport  Port=3
  Compare To Constant : simulink/Logic and Bit Operations/Compare To Constant  relop===  const=TRUE  OutDataTypeStr=boolean
  Compare To Constant1 : simulink/Logic and Bit Operations/Compare To Constant  relop===  const=TRUE  OutDataTypeStr=boolean
  Compare To Constant2 : simulink/Logic and Bit Operations/Compare To Constant  relop===  const=TRUE  OutDataTypeStr=boolean
  Logical Operator : Logic  Operator=OR  Inputs=3  AllPortsSameDT=off  OutDataTypeStr=boolean
  vehPreWarningAborCondFulfilled : Outport
  wires:
  vehPreWarningAborCond/1 -> Compare To Constant/1
  preWarningSuppArb/1 -> Compare To Constant1/1
  vehPreWarningFuncImpAborCond/1 -> Compare To Constant2/1
  Compare To Constant1/1 -> Logical Operator/2
  Compare To Constant/1 -> Logical Operator/1
  Compare To Constant2/1 -> Logical Operator/3
  Logical Operator/1 -> vehPreWarningAborCondFulfilled/1

== system TosAeb/FcwAebJa_Step_sys/ALG_AEBSystem/ALG_AEBController/AEB_StateMachineCalcApp/VehAEBStateMachine/PrefillStateMachine/AEBFuncFaultMap ==
  FCW_AEB_VehFctSts : Inport
  Compare To Constant : simulink/Logic and Bit Operations/Compare To Constant  relop===  const=CN_VSC_FCW_AEB_Error  OutDataTypeStr=boolean
  Fault : Outport
  wires:
  FCW_AEB_VehFctSts/1 -> Compare To Constant/1
  Compare To Constant/1 -> Fault/1

== system TosAeb/FcwAebJa_Step_sys/ALG_AEBSystem/ALG_AEBController/AEB_StateMachineCalcApp/VehAEBStateMachine/PrefillStateMachine/ActivationCondCalc ==
  vehPrefillSuppCond : Inport
  vehPrefillFuncImpSuppCond : Inport  Port=2
  vehPrefillBrkReadySuppArb : Inport  Port=3
  Compare To Constant : simulink/Logic and Bit Operations/Compare To Constant  relop===  const=FALSE  OutDataTypeStr=boolean
  Compare To Constant1 : simulink/Logic and Bit Operations/Compare To Constant  relop===  const=FALSE  OutDataTypeStr=boolean
  Compare To Constant2 : simulink/Logic and Bit Operations/Compare To Constant  relop===  const=FALSE  OutDataTypeStr=boolean
  Logical Operator : Logic  Inputs=3  AllPortsSameDT=off  OutDataTypeStr=boolean
  ActivationCondFulfilled : Outport
  wires:
  vehPrefillSuppCond/1 -> Compare To Constant/1
  vehPrefillFuncImpSuppCond/1 -> Compare To Constant1/1
  vehPrefillBrkReadySuppArb/1 -> Compare To Constant2/1
  Compare To Constant1/1 -> Logical Operator/2
  Compare To Constant/1 -> Logical Operator/1
  Compare To Constant2/1 -> Logical Operator/3
  Logical Operator/1 -> ActivationCondFulfilled/1  "ActivationCondFulfilled"

== system TosAeb/FcwAebJa_Step_sys/ALG_AEBSystem/ALG_AEBController/AEB_StateMachineCalcApp/VehAEBStateMachine/PrefillStateMachine/ActiveStateAborCondCalc ==
  vehPrefillAborCond : Inport
  prefillBrkReadyAborArb : Inport  Port=2
  vehPrefillFuncImpAborCond : Inport  Port=3
  brkImpOpcAborCond : Inport  Port=4
  Compare To Constant{,1..3} (4 alike) : simulink/Logic and Bit Operations/Compare To Constant  relop===  const=TRUE  OutDataTypeStr=boolean
  Logical Operator : Logic  Operator=OR  Inputs=4  AllPortsSameDT=off  OutDataTypeStr=boolean
  ActiveStateAborCondFulfilled : Outport
  wires:
  Logical Operator/1 -> ActiveStateAborCondFulfilled/1
  Compare To Constant2/1 -> Logical Operator/3
  Compare To Constant/1 -> Logical Operator/1
  Compare To Constant1/1 -> Logical Operator/2
  vehPrefillFuncImpAborCond/1 -> Compare To Constant2/1
  prefillBrkReadyAborArb/1 -> Compare To Constant1/1
  vehPrefillAborCond/1 -> Compare To Constant/1
  brkImpOpcAborCond/1 -> Compare To Constant3/1
  Compare To Constant3/1 -> Logical Operator/4

== system TosAeb/FcwAebJa_Step_sys/ALG_AEBSystem/ALG_AEBController/AEB_StateMachineCalcApp/VehFCTModStsArb/BrkArb/BrkAborArb ==
  note: BrkRelSuppAborCondArb
  vehAEBModSts : Inport
  Bus Selector : BusSelector  OutputSignals=vehAEBfullModSts,vehEBAModSts
  Compare To Constant2 : simulink/Logic and Bit Operations/Compare To Constant  relop===  const=ModSts.ActiveState  OutDataTypeStr=boolean
  Compare To Constant3 : simulink/Logic and Bit Operations/Compare To Constant  relop===  const=ModSts.ActiveState  OutDataTypeStr=boolean
  Constant : Constant  Value=FALSE  OutDataTypeStr=boolean
  Logical Operator : Logic  Operator=OR  AllPortsSameDT=off  OutDataTypeStr=boolean
  AEBpartAborArb : Outport
  AEBfullAborArb : Outport  Port=2
  EBAAborArb : Outport  Port=3
  wires:
  Logical Operator/1 -> AEBpartAborArb/1
  Compare To Constant2/1 -> Logical Operator/1
  Compare To Constant3/1 -> AEBfullAborArb/1, Logical Operator/2
  Bus Selector/2 -> Compare To Constant3/1  "<vehEBAModSts>"
  Bus Selector/1 -> Compare To Constant2/1  "<vehAEBfullModSts>"
  vehAEBModSts/1 -> Bus Selector/1
  Constant/1 -> EBAAborArb/1

== system TosAeb/FcwAebJa_Step_sys/ALG_AEBSystem/ALG_AEBController/AEB_StateMachineCalcApp/VehFCTModStsArb/BrkArb/BrkActvArb ==
  AEBpartModStsArbtrtn : Inport
  AEBfullModStsArbtrtn : Inport  Port=2
  EBAModStsArbtrtn : Inport  Port=3
  Compare To Constant : simulink/Logic and Bit Operations/Compare To Constant  relop===  const=ModSts.ActiveState  OutDataTypeStr=boolean
  Compare To Constant1 : simulink/Logic and Bit Operations/Compare To Constant  relop===  const=ModSts.ActiveState  OutDataTypeStr=boolean
  Compare To Constant2 : simulink/Logic and Bit Operations/Compare To Constant  relop===  const=ModSts.ActiveState  OutDataTypeStr=boolean
  Logical Operator : Logic  Operator=OR  Inputs=3  AllPortsSameDT=off  OutDataTypeStr=boolean
  brkActvCond : Outport
  wires:
  Compare To Constant2/1 -> Logical Operator/3
  Compare To Constant1/1 -> Logical Operator/2
  Compare To Constant/1 -> Logical Operator/1
  Logical Operator/1 -> brkActvCond/1
  AEBpartModStsArbtrtn/1 -> Compare To Constant/1
  AEBfullModStsArbtrtn/1 -> Compare To Constant1/1
  EBAModStsArbtrtn/1 -> Compare To Constant2/1

== system TosAeb/FcwAebJa_Step_sys/ALG_AEBSystem/ALG_AEBController/AEB_StateMachineCalcApp/VehFCTModStsArb/BrkArb/BrkSuppArb ==
  vehAEBModSts : Inport
  Bus Selector : BusSelector  OutputSignals=vehAEBfullModSts,vehEBAModSts
  Compare To Constant2 : simulink/Logic and Bit Operations/Compare To Constant  relop===  const=ModSts.ActiveState  OutDataTypeStr=boolean
  Compare To Constant6 : simulink/Logic and Bit Operations/Compare To Constant  relop===  const=ModSts.ActiveState  OutDataTypeStr=boolean
  Constant : Constant  Value=FALSE
  Logical Operator : Logic  Operator=OR  AllPortsSameDT=off  OutDataTypeStr=boolean
  AEBpartSuppArb : Outport
  AEBfullSuppArb : Outport  Port=2
  EBASuppArb : Outport  Port=3
  wires:
  Logical Operator/1 -> AEBpartSuppArb/1
  Bus Selector/2 -> Compare To Constant2/1  "<vehEBAModSts>"
  Bus Selector/1 -> Compare To Constant6/1  "<vehAEBfullModSts>"
  vehAEBModSts/1 -> Bus Selector/1
  Compare To Constant6/1 -> Logical Operator/1
  Compare To Constant2/1 -> AEBfullSuppArb/1, Logical Operator/2
  Constant/1 -> EBASuppArb/1

== system TosAeb/FcwAebJa_Step_sys/ALG_AEBSystem/ALG_AEBController/AEB_StateMachineCalcApp/VehFCTModStsArb/BrkArb/WarningModStsArbtrtn ==
  vehAEBModSts : Inport
  AEBpartAborArb : Inport  Port=2
  AEBfullAborArb : Inport  Port=3
  EBAAborArb : Inport  Port=4
  Bus Selector : BusSelector  OutputSignals=vehAEBpartModSts
  Bus Selector1 : BusSelector  OutputSignals=vehAEBfullModSts
  Bus Selector2 : BusSelector  OutputSignals=vehEBAModSts
  Compare To Constant : simulink/Logic and Bit Operations/Compare To Constant  relop=~=  const=ModSts.ActiveState  OutDataTypeStr=boolean
  Compare To Constant1 : simulink/Logic and Bit Operations/Compare To Constant  relop===  const=FALSE  OutDataTypeStr=boolean
  Compare To Constant2 : simulink/Logic and Bit Operations/Compare To Constant  relop=~=  const=ModSts.ActiveState  OutDataTypeStr=boolean
  Compare To Constant3 : simulink/Logic and Bit Operations/Compare To Constant  relop===  const=FALSE  OutDataTypeStr=boolean
  Compare To Constant4 : simulink/Logic and Bit Operations/Compare To Constant  relop=~=  const=ModSts.ActiveState  OutDataTypeStr=boolean
  Compare To Constant5 : simulink/Logic and Bit Operations/Compare To Constant  relop===  const=FALSE  OutDataTypeStr=boolean
  Constant : Constant  Value=ModSts.StandbyState
  Constant1 : Constant  Value=ModSts.StandbyState
  Constant2 : Constant  Value=ModSts.StandbyState
  Switch{,1..5} (6 alike) : Switch  Criteria=u2 ~= 0
  AEBpartModStsArb : Outport
  AEBfullModStsArb : Outport  Port=2
  EBAModStsArb : Outport  Port=3
  wires:
  Bus Selector/1 -> Switch/1, Switch1/1, Compare To Constant/1  "<vehAEBpartModSts>"
  Compare To Constant/1 -> Switch/2
  Switch1/1 -> Switch/3
  Compare To Constant1/1 -> Switch1/2
  AEBpartAborArb/1 -> Compare To Constant1/1
  Constant/1 -> Switch1/3
  Switch/1 -> AEBpartModStsArb/1
  Bus Selector1/1 -> Switch3/1, Switch2/1, Compare To Constant2/1  "<vehAEBfullModSts>"
  Compare To Constant2/1 -> Switch2/2
  AEBfullAborArb/1 -> Compare To Constant3/1
  Compare To Constant3/1 -> Switch3/2
  Constant1/1 -> Switch3/3
  Switch3/1 -> Switch2/3
  Switch2/1 -> AEBfullModStsArb/1
  Switch4/1 -> EBAModStsArb/1
  Switch5/1 -> Switch4/3
  Constant2/1 -> Switch5/3
  Compare To Constant5/1 -> Switch5/2
  EBAAborArb/1 -> Compare To Constant5/1
  Compare To Constant4/1 -> Switch4/2
  Bus Selector2/1 -> Compare To Constant4/1, Switch4/1, Switch5/1  "<vehEBAModSts>"
  vehAEBModSts/1 -> Bus Selector2/1, Bus Selector1/1, Bus Selector/1

== system TosAeb/FcwAebJa_Step_sys/ALG_AEBSystem/ALG_AEBController/AEB_StateMachineCalcApp/VehFCTModStsArb/BrkReadyArb/BrkReadyAborArb ==
  brkActvCond : Inport
  Compare To Constant : simulink/Logic and Bit Operations/Compare To Constant  relop===  const=TRUE  OutDataTypeStr=boolean
  Constant : Constant  Value=FALSE  OutDataTypeStr=boolean
  prefillBrkReadyAborArb : Outport
  ABABrkReadyAborArb : Outport  Port=2
  wires:
  Constant/1 -> ABABrkReadyAborArb/1
  brkActvCond/1 -> Compare To Constant/1
  Compare To Constant/1 -> prefillBrkReadyAborArb/1

== system TosAeb/FcwAebJa_Step_sys/ALG_AEBSystem/ALG_AEBController/AEB_StateMachineCalcApp/VehFCTModStsArb/BrkReadyArb/BrkReadyActvArb ==
  prefillModStsArb : Inport
  ABAModStsArb : Inport  Port=2
  Compare To Constant : simulink/Logic and Bit Operations/Compare To Constant  relop===  const=ModSts.ActiveState  OutDataTypeStr=boolean
  Compare To Constant1 : simulink/Logic and Bit Operations/Compare To Constant  relop===  const=ModSts.ActiveState  OutDataTypeStr=boolean
  Logical Operator : Logic  Operator=OR  AllPortsSameDT=off  OutDataTypeStr=boolean
  brkReadyActv : Outport
  wires:
  prefillModStsArb/1 -> Compare To Constant/1
  Compare To Constant/1 -> Logical Operator/1
  Compare To Constant1/1 -> Logical Operator/2
  Logical Operator/1 -> brkReadyActv/1
  ABAModStsArb/1 -> Compare To Constant1/1
